simulink model "TosAeb"  (R2021a)
settings: Solver=FixedStepDiscrete  StartTime=0.0  StopTime=inf  FixedStep=0.01  RelTol=1e-3
undefined symbols (not defined in the file or in its folder): ACCModSts, AccSts1, AEB_ObjectMovingOrientation, AEB_ObjectMovingState, CN_BTCActive, CN_CurrentGearR, CN_CurrentGearR_DHT, CN_PTCActive, CN_VDCActive, CN_VSC_EspOff, CN_VSC_FCW_AEB_Error, CN_VSC_FCW_AEB_NoDispay, CN_VSC_FCW_AEB_PerfmDgdtn, CN_VSC_FCW_AEB_TempUnAvlbl, CN_VSC_VehStandstill, CNaccelAvoidMax, CNaccelAvoidMin, CNbrakePedalPress, CNcomfortStrLeftAccelMax, CNcomfortStrLeftAccelMin, CNcomfortStrRightAccelMax, CNcomfortStrRightAccelMin, CNDrvDoorOpen, CNDrvModeEco, CNDrvModeNorm, CNDrvModeOther, CNDrvModeSport, CNDrvSBRreminder, CNNull, CNobjInvalidID, CNPassDoorOpen, CNPassSBRreminder, CNPEBSAccelNegInf, CNPEBSAccelNull, CNPEBSTimeInf, CNPEBSTimeNull, CNSTOPMAX, CNSTOPNULL, CNsubjWidth, CNSwtOFF, CNSwtON, CNTTCMAX, CNTTCNULL, CNVehConfigAEBCycNone, CNVehConfigAEBNone, CNVehConfigAEBPedNone, CNVehConfigFCWNone, CNVehConfigJANone, CollisionSeverity, FALSE, FCWSnvty, JACycObjCalcInfoDefaultParams, JAPedObjCalcInfoDefaultParams, JAVehObjCalcInfoDefaultParams, latCycObjCalcInfoDefaultParams, latPedObjCalcInfoDefaultParams, longObjCalcInfoDefaultParams, ModSts, movingStatus, ObjectType ... (+1112)
libraries: simulink x3031, AEBSFunctionLib x50, Ta_Library x33, AEBSStateMachineLib x23, Mylibrary x12, simulink_extras x7, ObjSelect_lib x3, dspstat3 x2
(part 62 of 102)

== system TosAeb/FcwAebJa_Step_sys/ALG_AEBSystem/ALG_AEBController/AEB_LatObjInfoCalcApp/LatCycObjCalc/LatCycCollisonAssessInfoCalc/LatCycCollisonAssessInfoCalc/DistBrkCondCalc/CycDistBrakePartCalc/DistPhase3Calc ==
  egoStopFlg : Inport
  tJerkWork : Inport  Port=2  OutDataTypeStr=single
  tPhase2 : Inport  Port=3  OutDataTypeStr=single
  tPhase1 : Inport  Port=4  OutDataTypeStr=single
  velDelta : Inport  Port=5  OutDataTypeStr=single
  subjVel : Inport  Port=6
  subjLongAccelFilt : Inport  Port=7  OutDataTypeStr=single
  Kjerk : Inport  Port=8  OutDataTypeStr=single
  accelMax : Inport  Port=9  OutDataTypeStr=single
  Bus Creator : BusCreator  Inputs=8
  Bus Selector : BusSelector  OutputSignals=subjVel,subjLongAccelFilt,tJerkWork,Kjerk,accelMax,velDelta
  Bus Selector1 : BusSelector  OutputSignals=subjVel,subjLongAccelFilt,Kjerk,tPhase1,tPhase2,accelMax
  If : If  IfExpression=u1
  Merge : Merge
  distPhase3Calc : Subsystem
  tPhase3Calc : Subsystem  TreatAsAtomicUnit=on
  tPhase3Calc1 : Subsystem  TreatAsAtomicUnit=on
  distPhase3 : Outport
  wires:
  Bus Selector1/1 -> distPhase3Calc/2(subjVel)  "<subjVel>"
  Bus Selector1/2 -> distPhase3Calc/3(subjLongAccelFilt)  "<subjLongAccelFilt>"
  Bus Selector1/3 -> distPhase3Calc/4(Kjerk)  "<Kjerk>"
  Bus Selector1/4 -> distPhase3Calc/5(tPhase1)  "<tPhase1>"
  Bus Selector1/5 -> distPhase3Calc/6(tPhase2)  "<tPhase2>"
  Bus Selector1/6 -> distPhase3Calc/7(accelMax)  "<accelMax>"
  Bus Selector/6 -> tPhase3Calc/6(velDelta)  "<velDelta>"
  Bus Selector/1 -> tPhase3Calc/1(subjVel)  "<subjVel>"
  Bus Selector/5 -> tPhase3Calc/5(accelMax)  "<accelMax>"
  Bus Selector/2 -> tPhase3Calc/2(subjLongAccelFilt)  "<subjLongAccelFilt>"
  Bus Selector/4 -> tPhase3Calc/4(Kjerk)  "<Kjerk>"
  Bus Selector/3 -> tPhase3Calc/3(tJerkWork)  "<tJerkWork>"
  Bus Creator/1 -> Bus Selector/1, Bus Selector1/1
  velDelta/1 -> Bus Creator/4  "velDelta"
  tPhase2/1 -> Bus Creator/3  "tPhase2"
  tPhase1/1 -> Bus Creator/2  "tPhase1"
  tJerkWork/1 -> Bus Creator/1  "tJerkWork"
  subjVel/1 -> Bus Creator/5  "subjVel"
  subjLongAccelFilt/1 -> Bus Creator/6  "subjLongAccelFilt"
  Kjerk/1 -> Bus Creator/7  "Kjerk"
  accelMax/1 -> Bus Creator/8  "accelMax"
  distPhase3Calc/1(distPhase3) -> distPhase3/1
  Merge/1 -> distPhase3Calc/1(tPhase3)
  tPhase3Calc/1(tPhase3) -> Merge/2
  tPhase3Calc1/1(tPhase3) -> Merge/1
  If/2 -> tPhase3Calc/Action
  egoStopFlg/1 -> If/1
  If/1 -> tPhase3Calc1/Action

== system TosAeb/FcwAebJa_Step_sys/ALG_AEBSystem/ALG_AEBController/AEB_LatObjInfoCalcApp/LatCycObjCalc/LatCycCollisonAssessInfoCalc/LatCycCollisonAssessInfoCalc/DistBrkCondCalc/CycDistBrakePartCalc/distBrakeCalc ==
  distPhase1 : Inport  OutDataTypeStr=single
  distPhase2 : Inport  Port=2  OutDataTypeStr=single
  distPhase3 : Inport  Port=3  OutDataTypeStr=single
  Add : Sum  Inputs=3  OutDataTypeStr=single
  distBrake : Outport
  wires:
  Add/1 -> distBrake/1
  distPhase3/1 -> Add/3
  distPhase1/1 -> Add/1
  distPhase2/1 -> Add/2

== system TosAeb/FcwAebJa_Step_sys/ALG_AEBSystem/ALG_AEBController/AEB_LatObjInfoCalcApp/LatCycObjCalc/LatCycCollisonAssessInfoCalc/LatCycCollisonAssessInfoCalc/DistBrkCondCalc/distBraking4FullBrakeCalc1/DistPhase1Calc ==
  subjlongAccelCorr : Inport  OutDataTypeStr=single
  tdelay : Inport  Port=2  OutDataTypeStr=single
  subjVel : Inport  Port=3
  Add : Sum  OutDataTypeStr=single
  Gain : Gain  Gain=0.5  OutDataTypeStr=single
  Math Function : Math  Operator=square  SignedPower=on  OutDataTypeStr=single  SaturateOnIntegerOverflow=on
  Product : Product  OutDataTypeStr=single
  Product1 : Product  OutDataTypeStr=single
  distPhase1 : Outport
  timeWorkPhase1 : Outport  Port=2
  wires:
  Gain/1 -> Add/1
  Product/1 -> Add/2
  Add/1 -> distPhase1/1
  Product1/1 -> Gain/1
  subjlongAccelCorr/1 -> Product1/1
  Math Function/1 -> Product1/2
  subjVel/1 -> Product/2
  tdelay/1 -> Math Function/1, timeWorkPhase1/1, Product/1

== system TosAeb/FcwAebJa_Step_sys/ALG_AEBSystem/ALG_AEBController/AEB_LatObjInfoCalcApp/LatCycObjCalc/LatCycCollisonAssessInfoCalc/LatCycCollisonAssessInfoCalc/DistBrkCondCalc/distBraking4FullBrakeCalc1/DistPhase2Calc ==
  note: 增加输出接口4
  accelMax : Inport  OutDataTypeStr=single
  accelWorkPhase1 : Inport  Port=2  OutDataTypeStr=single
  Kjerk : Inport  Port=3  OutDataTypeStr=single
  subjVel : Inport  Port=4
  distPhase2Calc : Subsystem
  tWorkPhase2Calc : Subsystem
  distPhase2 : Outport
  Phase2EgoStopFlg : Outport  Port=2
  tWorkPhase2 : Outport  Port=3
  wires:
  [subjLongAccelCorr] -> distPhase2Calc/4(subjLongAccelCorr)
  [Kjerk] -> distPhase2Calc/3(Kjerk)
  accelWorkPhase1/1 -> [subjLongAccelCorr], tWorkPhase2Calc/2(accelWorkPhase1)
  accelMax/1 -> tWorkPhase2Calc/1(accelMax)
  Kjerk/1 -> [Kjerk], tWorkPhase2Calc/3(Kjerk)
  tWorkPhase2Calc/2(tWorkPhase2) -> tWorkPhase2/1, distPhase2Calc/1(tWorkPhase2)
  subjVel/1 -> tWorkPhase2Calc/4(subjVel), distPhase2Calc/2(subjVel)
  distPhase2Calc/1(distPhase2) -> distPhase2/1
  tWorkPhase2Calc/1(Phase2EgoStopFlg) -> Phase2EgoStopFlg/1

== system TosAeb/FcwAebJa_Step_sys/ALG_AEBSystem/ALG_AEBController/AEB_LatObjInfoCalcApp/LatCycObjCalc/LatCycCollisonAssessInfoCalc/LatCycCollisonAssessInfoCalc/DistBrkCondCalc/distBraking4FullBrakeCalc1/brkSysAtributeCalc ==
  subjVel : Inport
  subLongAccel : Inport  Port=2  OutDataTypeStr=single
  Bus Creator : BusCreator  Inputs=5
  Compare To Constant1 : simulink/Logic and Bit Operations/Compare To Constant  relop=>  const=11.94  OutDataTypeStr=boolean
  Constant : Constant  Value=0.5  OutDataTypeStr=single
  Constant2 : Constant  Value=-45  OutDataTypeStr=single
  Constant3 : Constant  Value=-10.5  OutDataTypeStr=single
  Constant4 : Constant  Value=-6.94  OutDataTypeStr=single
  Constant5 : Constant  Value=-11.11  OutDataTypeStr=single
  Min : MinMax  Function=max  Inputs=2  OutDataTypeStr=single
  Switch1 : Switch  Criteria=u2 ~= 0  OutDataTypeStr=single
  brkSysAtribute : Outport
  wires:
  Constant2/1 -> Bus Creator/2  "Kjerk"
  Bus Creator/1 -> brkSysAtribute/1
  Compare To Constant1/1 -> Switch1/2
  subjVel/1 -> Compare To Constant1/1
  Constant4/1 -> Switch1/1
  Constant5/1 -> Switch1/3
  Constant/1 -> Bus Creator/1  "tdelay"
  Constant3/1 -> Min/2, Bus Creator/4  "accelMax"
  Switch1/1 -> Bus Creator/5  "velReduceMax"
  subLongAccel/1 -> Min/1
  Min/1 -> Bus Creator/3  "subjLongAccelCorr"

== system TosAeb/FcwAebJa_Step_sys/ALG_AEBSystem/ALG_AEBController/AEB_LatObjInfoCalcApp/LatCycObjCalc/LatCycCollisonAssessInfoCalc/LatCycCollisonAssessInfoCalc/DistBrkCondCalc/distBraking4FullBrakeCalc1/distPhase3Calc ==
  Phase2EgoStopFlg : Inport
  tWorkPhase2 : Inport  Port=2  OutDataTypeStr=single
  subjLongAccelCorr : Inport  Port=3  OutDataTypeStr=single
  Kjerk : Inport  Port=4  OutDataTypeStr=single
  accelWorkPhase1 : Inport  Port=5  OutDataTypeStr=single
  velMaxReduce : Inport  Port=6  OutDataTypeStr=single
  accelMax : Inport  Port=7  OutDataTypeStr=single
  tWorkPhase1 : Inport  Port=8  OutDataTypeStr=single
  EgoStopped : Subsystem  TreatAsAtomicUnit=on
  If : If  IfExpression=u1
  Merge : Merge
  distPhase3Calc : Subsystem  TreatAsAtomicUnit=on
  distPhase3 : Outport
  wires:
  tWorkPhase1/1 -> distPhase3Calc/7(tWorkPhase1)
  accelMax/1 -> distPhase3Calc/6(accelMax)
  velMaxReduce/1 -> distPhase3Calc/5(velMaxReduce)
  accelWorkPhase1/1 -> distPhase3Calc/3(accelWorkPhase1)
  subjLongAccelCorr/1 -> distPhase3Calc/4(subjLongAccelCorr)
  tWorkPhase2/1 -> distPhase3Calc/2(tWorkPhase2)
  Kjerk/1 -> distPhase3Calc/1(Kjerk)
  Phase2EgoStopFlg/1 -> If/1
  If/2 -> distPhase3Calc/Action
  EgoStopped/1(distPhase3) -> Merge/1
  If/1 -> EgoStopped/Action
  Merge/1 -> distPhase3/1
  distPhase3Calc/1(distPhase3) -> Merge/2

== system TosAeb/FcwAebJa_Step_sys/ALG_AEBSystem/ALG_AEBController/AEB_LatObjInfoCalcApp/LatCycObjCalc/LatCycCollisonAssessInfoCalc/LatCycCollisonAssessInfoCalc/DistBrkCondCalc/distBraking4FullBrakeCalc1/distanceTriggerBrakingCalc ==
  distPhase1 : Inport  OutDataTypeStr=single
  distPhase2 : Inport  Port=2  OutDataTypeStr=single
  distPhase3 : Inport  Port=3  OutDataTypeStr=single
  Add : Sum  Inputs=+++  OutDataTypeStr=single
  DistBraking4FullBrake : Outport
  wires:
  Add/1 -> DistBraking4FullBrake/1
  distPhase3/1 -> Add/3
  distPhase2/1 -> Add/2
  distPhase1/1 -> Add/1

== system TosAeb/FcwAebJa_Step_sys/ALG_AEBSystem/ALG_AEBController/AEB_LatObjInfoCalcApp/LatCycObjCalc/LatCycCollisonAssessInfoCalc/LatCycCollisonAssessInfoCalc/DistBrkCondCalc/distBraking4PartBrakeCalc1/DistPhase1Calc ==
  subjlongAccelCorr : Inport  OutDataTypeStr=single
  tdelay : Inport  Port=2  OutDataTypeStr=single
  subjVel : Inport  Port=3
  Add : Sum  OutDataTypeStr=single
  Gain : Gain  Gain=0.5  OutDataTypeStr=single
  Math Function : Math  Operator=square  SignedPower=on  OutDataTypeStr=single  SaturateOnIntegerOverflow=on
  Product : Product  OutDataTypeStr=single
  Product1 : Product  OutDataTypeStr=single
  distPhase1 : Outport
  timeWorkPhase1 : Outport  Port=2
  wires:
  tdelay/1 -> Product/1, timeWorkPhase1/1, Math Function/1
  subjVel/1 -> Product/2
  Math Function/1 -> Product1/2
  subjlongAccelCorr/1 -> Product1/1
  Product1/1 -> Gain/1
  Add/1 -> distPhase1/1
  Product/1 -> Add/2
  Gain/1 -> Add/1

== system TosAeb/FcwAebJa_Step_sys/ALG_AEBSystem/ALG_AEBController/AEB_LatObjInfoCalcApp/LatCycObjCalc/LatCycCollisonAssessInfoCalc/LatCycCollisonAssessInfoCalc/DistBrkCondCalc/distBraking4PartBrakeCalc1/DistPhase2Calc ==
  note: 增加输出接口4
  accelMax : Inport  OutDataTypeStr=single
  accelWorkPhase1 : Inport  Port=2  OutDataTypeStr=single
  Kjerk : Inport  Port=3  OutDataTypeStr=single
  subjVel : Inport  Port=4
  distPhase2Calc : Subsystem
  tWorkPhase2Calc : Subsystem
  distPhase2 : Outport
  Phase2EgoStopFlg : Outport  Port=2
  tWorkPhase2 : Outport  Port=3
  wires:
  tWorkPhase2Calc/1(Phase2EgoStopFlg) -> Phase2EgoStopFlg/1
  distPhase2Calc/1(distPhase2) -> distPhase2/1
  subjVel/1 -> distPhase2Calc/2(subjVel), tWorkPhase2Calc/4(subjVel)
  tWorkPhase2Calc/2(tWorkPhase2) -> distPhase2Calc/1(tWorkPhase2), tWorkPhase2/1
  Kjerk/1 -> tWorkPhase2Calc/3(Kjerk), [Kjerk]
  accelMax/1 -> tWorkPhase2Calc/1(accelMax)
  accelWorkPhase1/1 -> tWorkPhase2Calc/2(accelWorkPhase1), [subjLongAccelCorr]
  [Kjerk] -> distPhase2Calc/3(Kjerk)
  [subjLongAccelCorr] -> distPhase2Calc/4(subjLongAccelCorr)

== system TosAeb/FcwAebJa_Step_sys/ALG_AEBSystem/ALG_AEBController/AEB_LatObjInfoCalcApp/LatCycObjCalc/LatCycCollisonAssessInfoCalc/LatCycCollisonAssessInfoCalc/DistBrkCondCalc/distBraking4PartBrakeCalc1/brkSysAtributeCalc ==
  subjVel : Inport
  subLongAccel : Inport  Port=2  OutDataTypeStr=single
  Bus Creator : BusCreator  Inputs=5
  Compare To Constant1 : simulink/Logic and Bit Operations/Compare To Constant  relop=>  const=11.94  OutDataTypeStr=boolean
  Constant : Constant  Value=0.5  OutDataTypeStr=single
  Constant2 : Constant  Value=-45  OutDataTypeStr=single
  Constant3 : Constant  Value=-5.75  OutDataTypeStr=single
  Constant4 : Constant  Value=-6.94  OutDataTypeStr=single
  Constant5 : Constant  Value=-8.3  OutDataTypeStr=single
  Min : MinMax  Function=max  Inputs=2  OutDataTypeStr=single
  Switch1 : Switch  Criteria=u2 ~= 0  OutDataTypeStr=single
  brkSysAtribute : Outport
  wires:
  Min/1 -> Bus Creator/3  "subjLongAccelCorr"
  subLongAccel/1 -> Min/1
  Switch1/1 -> Bus Creator/5  "velReduceMax"
  Constant3/1 -> Bus Creator/4, Min/2  "accelMax"
  Constant/1 -> Bus Creator/1  "tdelay"
  Constant5/1 -> Switch1/3
  Constant4/1 -> Switch1/1
  subjVel/1 -> Compare To Constant1/1
  Compare To Constant1/1 -> Switch1/2
  Bus Creator/1 -> brkSysAtribute/1
  Constant2/1 -> Bus Creator/2  "Kjerk"

== system TosAeb/FcwAebJa_Step_sys/ALG_AEBSystem/ALG_AEBController/AEB_LatObjInfoCalcApp/LatCycObjCalc/LatCycCollisonAssessInfoCalc/LatCycCollisonAssessInfoCalc/DistBrkCondCalc/distBraking4PartBrakeCalc1/distPhase3Calc ==
  Phase2EgoStopFlg : Inport
  tWorkPhase2 : Inport  Port=2  OutDataTypeStr=single
  subjLongAccelCorr : Inport  Port=3  OutDataTypeStr=single
  Kjerk : Inport  Port=4  OutDataTypeStr=single
  accelWorkPhase1 : Inport  Port=5  OutDataTypeStr=single
  velMaxReduce : Inport  Port=6  OutDataTypeStr=single
  accelMax : Inport  Port=7  OutDataTypeStr=single
  tWorkPhase1 : Inport  Port=8  OutDataTypeStr=single
  EgoStopped : Subsystem  TreatAsAtomicUnit=on
  If : If  IfExpression=u1
  Merge : Merge
  distPhase3Calc : Subsystem  TreatAsAtomicUnit=on
  distPhase3 : Outport
  wires:
  distPhase3Calc/1(distPhase3) -> Merge/2
  Merge/1 -> distPhase3/1
  If/1 -> EgoStopped/Action
  EgoStopped/1(distPhase3) -> Merge/1
  If/2 -> distPhase3Calc/Action
  Phase2EgoStopFlg/1 -> If/1
  Kjerk/1 -> distPhase3Calc/1(Kjerk)
  tWorkPhase2/1 -> distPhase3Calc/2(tWorkPhase2)
  subjLongAccelCorr/1 -> distPhase3Calc/4(subjLongAccelCorr)
  accelWorkPhase1/1 -> distPhase3Calc/3(accelWorkPhase1)
  velMaxReduce/1 -> distPhase3Calc/5(velMaxReduce)
  accelMax/1 -> distPhase3Calc/6(accelMax)
  tWorkPhase1/1 -> distPhase3Calc/7(tWorkPhase1)

== system TosAeb/FcwAebJa_Step_sys/ALG_AEBSystem/ALG_AEBController/AEB_LatObjInfoCalcApp/LatCycObjCalc/LatCycCollisonAssessInfoCalc/LatCycCollisonAssessInfoCalc/DistBrkCondCalc/distBraking4PartBrakeCalc1/distanceTriggerBrakingCalc ==
  distPhase1 : Inport  OutDataTypeStr=single
  distPhase2 : Inport  Port=2  OutDataTypeStr=single
  distPhase3 : Inport  Port=3  OutDataTypeStr=single
  Add : Sum  Inputs=+++  OutDataTypeStr=single
  distBraking4PartBrake : Outport
  wires:
  distPhase1/1 -> Add/1
  distPhase2/1 -> Add/2
  distPhase3/1 -> Add/3
  Add/1 -> distBraking4PartBrake/1

== system TosAeb/FcwAebJa_Step_sys/ALG_AEBSystem/ALG_AEBController/AEB_LatObjInfoCalcApp/LatCycObjCalc/LatCycCollisonAssessInfoCalc/LatCycCollisonAssessInfoCalc/DistBrkCondCalc/distSecurCalc/If Action Subsystem ==
  subjVel : Inport
  Action Port : ActionPort  ActionPortLabel=else
  1-D Lookup Table : Lookup_n-D  NumberOfTableDimensions=1  BreakpointsForDimension1=P_AEB_LatCycCA_SubjVelDxSecureUnder40KphX  BeginIndexSearchUsingPreviousIndexResult=on  Table=P_AEB_LatCycCA_DxSecureY  ExtrapMethod=Clip  InternalRulePriority=Speed  RndMeth=Simplest  InputSameDT=on
  distSecure : Outport
  wires:
  1-D Lookup Table/1 -> distSecure/1
  subjVel/1 -> 1-D Lookup Table/1

== system TosAeb/FcwAebJa_Step_sys/ALG_AEBSystem/ALG_AEBController/AEB_LatObjInfoCalcApp/LatCycObjCalc/LatCycCollisonAssessInfoCalc/LatCycCollisonAssessInfoCalc/DistBrkCondCalc/distSecurCalc/If Action Subsystem1 ==
  Action Port : ActionPort  ActionPortLabel=if(u1)
  Constant : Constant  Value=CNNull
  distSecure : Outport
  wires:
  Constant/1 -> distSecure/1

== system TosAeb/FcwAebJa_Step_sys/ALG_AEBSystem/ALG_AEBController/AEB_LatObjInfoCalcApp/LatCycObjCalc/LatCycObjCalc/latCycObjInfoCalc/ObjColiisionRiskProbCalc/CollisionRiskProbCalc/CollisionRiskProbCalc ==
  dxReginEgoRUCorr : Inport
  dyReginEgoRUCorr : Inport  Port=2
  dyReginEgoLLCorr : Inport  Port=3
  dxReginEgoLLCorr : Inport  Port=4
  dxReginObjRUCorr : Inport  Port=5
  dyReginObjRUCorr : Inport  Port=6
  dxReginObjLLCorr : Inport  Port=7
  dyReginObjLLCorr : Inport  Port=8
  Bus Creator : BusCreator  Inputs=8
  Bus Selector : BusSelector  OutputSignals=dyReginEgoRUCorr,dyReginEgoLLCorr,dyReginObjRUCorr,dyReginObjLLCorr
  Bus Selector1 : BusSelector  OutputSignals=dxReginEgoRUCorr,dxReginEgoLLCorr,dxReginObjRUCorr,dxReginObjLLCorr
  Bus Selector2 : BusSelector  OutputSignals=dxReginObjLLCorr,dyReginObjRUCorr,dyReginObjLLCorr
  CriticalityPointCalc : Subsystem
  dxOverlapFlow : Stateflow chart  TreatAsAtomicUnit=on
  dyOverlapFlow : Stateflow chart  TreatAsAtomicUnit=on
  pedCollisionRiskProbCalc : Subsystem
  dxPreCollison : Outport
  dyPreCollison : Outport  Port=2
  collisionRiskProb : Outport  Port=3
  dyCollisionRiskProb : Outport  Port=4
  dxCollisionRiskProb : Outport  Port=5
  wires:
  pedCollisionRiskProbCalc/3(dxCollisionRiskProb) -> dxCollisionRiskProb/1
  pedCollisionRiskProbCalc/2(dyCollisionRiskProb) -> dyCollisionRiskProb/1
  Bus Selector1/4 -> dxOverlapFlow/4(dxReginObjLLCorr)  "<dxReginObjLLCorr>"
  Bus Selector1/3 -> dxOverlapFlow/3(dxReginObjRUCorr)  "<dxReginObjRUCorr>"
  Bus Selector/4 -> dyOverlapFlow/4(dyReginObjLLCorr)  "<dyReginObjLLCorr>"
  Bus Selector/3 -> dyOverlapFlow/3(dyReginObjRUCorr)  "<dyReginObjRUCorr>"
  CriticalityPointCalc/2(dyPreCollison) -> dyPreCollison/1
  CriticalityPointCalc/1(dxPreCollison) -> dxPreCollison/1
  Bus Selector2/3 -> CriticalityPointCalc/3(dyReginObjLLCorr)  "<dyReginObjLLCorr>"
  Bus Selector2/2 -> CriticalityPointCalc/2(dyReginObjRUCorr)  "<dyReginObjRUCorr>"
  Bus Selector2/1 -> CriticalityPointCalc/1(dxReginObjLLCorr)  "<dxReginObjLLCorr>"
  Bus Selector1/2 -> dxOverlapFlow/2(dxReginEgoLLCorr)  "<dxReginEgoLLCorr>"
  Bus Selector1/1 -> dxOverlapFlow/1(dxReginEgoRUCorr)  "<dxReginEgoRUCorr>"
  Bus Selector/2 -> dyOverlapFlow/2(dyReginEgoLLCorr)  "<dyReginEgoLLCorr>"
  Bus Selector/1 -> dyOverlapFlow/1(dyReginEgoRUCorr)  "<dyReginEgoRUCorr>"
  Bus Creator/1 -> Bus Selector2/1, Bus Selector/1, Bus Selector1/1
  dyReginObjLLCorr/1 -> Bus Creator/8  "dyReginObjLLCorr"
  dxReginObjLLCorr/1 -> Bus Creator/7  "dxReginObjLLCorr"
  dyReginObjRUCorr/1 -> Bus Creator/6  "dyReginObjRUCorr"
  dxReginEgoRUCorr/1 -> Bus Creator/1  "dxReginEgoRUCorr"
  dxReginObjRUCorr/1 -> Bus Creator/5  "dxReginObjRUCorr"
  dxReginEgoLLCorr/1 -> Bus Creator/4  "dxReginEgoLLCorr"
  dyReginEgoRUCorr/1 -> Bus Creator/2  "dyReginEgoRUCorr"
  dyReginEgoLLCorr/1 -> Bus Creator/3  "dyReginEgoLLCorr"
  pedCollisionRiskProbCalc/1(collisionRiskProb) -> collisionRiskProb/1
  dxOverlapFlow/2(dxOverlapLimit) -> pedCollisionRiskProbCalc/4(dxOverlapLimit)
  dxOverlapFlow/1(dxOverlap) -> pedCollisionRiskProbCalc/3(dxOverlap)
  dyOverlapFlow/2(dyOverlapLimit) -> pedCollisionRiskProbCalc/2(dyOverlapLimit)
  dyOverlapFlow/1(dyOverlap) -> pedCollisionRiskProbCalc/1(dyOverlap)

== system TosAeb/FcwAebJa_Step_sys/ALG_AEBSystem/ALG_AEBController/AEB_LatObjInfoCalcApp/LatCycObjCalc/LatCycObjCalc/latCycObjInfoCalc/ObjColiisionRiskProbCalc/CollisionRiskProbCalc/EgoReginCalc ==
  tPredTime : Inport
  subjVel : Inport  Port=2
  Abs : Abs
  Add : Sum
  Add1 : Sum  Inputs=+-
  Constant : Constant  Value=P_Global_DxvRearBumper_m
  Constant1 : Constant  Value=P_Global_VehicleWidthMin_m
  Constant2 : Constant  Value=P_Global_DxvFrontBumper_m
  Gain : Gain  Gain=-0.5
  Gain1 : Gain  Gain=0.5
  Product : Product
  dxReginEgoRU : Outport
  dyReginEgoRU : Outport  Port=2
  dxReginEgoLL : Outport  Port=3
  dyReginEgoLL : Outport  Port=4
  wires:
  subjVel/1 -> Product/2
  tPredTime/1 -> Product/1
  Add1/1 -> dxReginEgoLL/1
  Constant/1 -> Abs/1
  Gain1/1 -> dyReginEgoLL/1
  Gain/1 -> dyReginEgoRU/1
  Constant1/1 -> Gain/1, Gain1/1
  Add/1 -> dxReginEgoRU/1
  Constant2/1 -> Add/2
  Product/1 -> Add1/1, Add/1
  Abs/1 -> Add1/2

== system TosAeb/FcwAebJa_Step_sys/ALG_AEBSystem/ALG_AEBController/AEB_LatObjInfoCalcApp/LatCycObjCalc/LatCycObjCalc/latCycObjInfoCalc/ObjColiisionRiskProbCalc/CollisionRiskProbCalc/EgoReginCorrCalc ==
  dxReginEgoRU : Inport
  dyReginEgoRU : Inport  Port=2
  dxReginEgoLL : Inport  Port=3
  dyReginEgoLL : Inport  Port=4
  Add : Sum  OutDataTypeStr=single
  Add1 : Sum  Inputs=+-  OutDataTypeStr=single
  Add2 : Sum  OutDataTypeStr=single
  ObjectLength : Constant  Value=0.5  OutDataTypeStr=single
  ObjectWidth : Constant  Value=1.8  OutDataTypeStr=single
  dxReginEgoRUCorr : Outport
  dyReginEgoRUCorr : Outport  Port=2
  dyReginEgoLLCorr : Outport  Port=3
  dxReginEgoLLCorr : Outport  Port=4
  wires:
  ObjectLength/1 -> Add/1
  dxReginEgoRU/1 -> Add/2
  Add2/1 -> dyReginEgoLLCorr/1
  dyReginEgoLL/1 -> Add2/2
  dxReginEgoLL/1 -> dxReginEgoLLCorr/1
  Add1/1 -> dyReginEgoRUCorr/1
  ObjectWidth/1 -> Add1/2, Add2/1
  dyReginEgoRU/1 -> Add1/1
  Add/1 -> dxReginEgoRUCorr/1

== system TosAeb/FcwAebJa_Step_sys/ALG_AEBSystem/ALG_AEBController/AEB_LatObjInfoCalcApp/LatCycObjCalc/LatCycObjCalc/latCycObjInfoCalc/ObjColiisionRiskProbCalc/CollisionRiskProbCalc/ObjAbsInfoCalc ==
  vx : Inport
  vy : Inport  Port=2
  dy : Inport  Port=3
  dxFrontBumper : Inport  Port=4
  subjYawRate : Inport  Port=5
  subjVel : Inport  Port=6
  Bus Creator1 : BusCreator  Inputs=2
  RadicalRelevantCalc : Subsystem
  RadicalToXYTrans : Subsystem
  XYToRadicalTrans : Subsystem
  sincosCoefCalc : Subsystem
  velAbsObjCorr : Outport
  wires:
  RadicalToXYTrans/1(velAbsObjCorr) -> velAbsObjCorr/1
  RadicalRelevantCalc/2(objAngleToRear) -> sincosCoefCalc/1(angle)  "objAngleToRear"
  XYToRadicalTrans/1(velRadicalXY) -> RadicalToXYTrans/2(velRadicalXY)
  vy/1 -> Bus Creator1/2  "vyRelTargetObjCalc"
  Bus Creator1/1 -> XYToRadicalTrans/1(LongLatVelRel)  "LongLatVelRel"
  dxFrontBumper/1 -> RadicalRelevantCalc/2(dxTargetObjCalc)
  subjVel/1 -> RadicalToXYTrans/1(subjVel)
  vx/1 -> Bus Creator1/1  "vxRelTargetObjCalc"
  sincosCoefCalc/1(sincosCoef) -> RadicalToXYTrans/3(sincosCoef), XYToRadicalTrans/3(sincosCoef)
  dy/1 -> RadicalRelevantCalc/1(dyTargetObjCalc)
  subjYawRate/1 -> RadicalRelevantCalc/3(subjYawRate)
  RadicalRelevantCalc/1(radialVelRel) -> XYToRadicalTrans/2(radialVelRel)  "radialVelRel"

== system TosAeb/FcwAebJa_Step_sys/ALG_AEBSystem/ALG_AEBController/AEB_LatObjInfoCalcApp/LatCycObjCalc/LatCycObjCalc/latCycObjInfoCalc/ObjColiisionRiskProbCalc/CollisionRiskProbCalc/ObjReginCorrCalc ==
  velAbsObjCorr : Inport
  tPredTime : Inport  Port=2
  ObjectTypeCameraConfirmed : Inport  Port=3
  ObjectWidth : Inport  Port=4
  ObjectYawAngle : Inport  Port=5
  ObjectMovingOrientation : Inport  Port=6
  ObjectLength : Inport  Port=7
  dx : Inport  Port=8
  dy : Inport  Port=9
  vxAbsObj : Inport  Port=10
  vy : Inport  Port=11
  AccelXYMaxMinCorrCalc : Subsystem
  CycMaxAccelDecelCalc : Subsystem
  ObjFrameCalc : Subsystem
  ObjLengthWidthCalc : Subsystem
  ObjLengthWidthCalc1 : Subsystem  Commented=on
  ObjReginCorrCalc : Subsystem
  dxReginObjRUCorr : Outport
  dyReginObjRUCorr : Outport  Port=2
  dxReginObjLLCorr : Outport  Port=3
  dyReginObjLLCorr : Outport  Port=4
  wires:
  ObjReginCorrCalc/4(dyReginObjLLCorr) -> dyReginObjLLCorr/1
  ObjReginCorrCalc/3(dxReginObjLLCorr) -> dxReginObjLLCorr/1
  ObjReginCorrCalc/2(dyReginObjRUCorr) -> dyReginObjRUCorr/1
  ObjReginCorrCalc/1(dxReginObjRUCorr) -> dxReginObjRUCorr/1
  ObjLengthWidthCalc/2(ObjWidthCorr) -> ObjFrameCalc/4(ObjWidthCorr)
  ObjLengthWidthCalc/1(ObjLengthCorr) -> ObjFrameCalc/3(ObjLengthCorr)
  ObjectLength/1 -> ObjLengthWidthCalc/2(ObjectLength)
  ObjectYawAngle/1 -> ObjLengthWidthCalc/4(ObjectYawAngle)
  ObjectWidth/1 -> ObjLengthWidthCalc/3(ObjectWidth)
  dy/1 -> ObjFrameCalc/2(dy)
  dx/1 -> ObjFrameCalc/1(dx)
  ObjFrameCalc/4(dyMin) -> ObjReginCorrCalc/7(dyMin)
  ObjFrameCalc/2(dxMin) -> ObjReginCorrCalc/5(dxMin)
  AccelXYMaxMinCorrCalc/2(AccelYMaxMinCorr) -> ObjReginCorrCalc/2(AccelYMaxMinCorr)
  ObjFrameCalc/3(dyMax) -> ObjReginCorrCalc/6(dyMax)
  ObjFrameCalc/1(dxMax) -> ObjReginCorrCalc/4(dxMax)
  CycMaxAccelDecelCalc/1(maxAccelDecel) -> AccelXYMaxMinCorrCalc/1(maxAccelDecel)
  velAbsObjCorr/1 -> ObjReginCorrCalc/3(velAbsTargetCorr), AccelXYMaxMinCorrCalc/2(velAbsTargetCorr)
  AccelXYMaxMinCorrCalc/1(AccelXMaxMinCorr) -> ObjReginCorrCalc/1(AccelXMaxMinCorr)
  tPredTime/1 -> ObjReginCorrCalc/8(tPredTime)
  vxAbsObj/1 -> ObjReginCorrCalc/9(vxAbsObj)
  vy/1 -> ObjReginCorrCalc/10(vy)
  ObjectMovingOrientation/1 -> ObjLengthWidthCalc/5(ObjectMovingOrientation)
  ObjectTypeCameraConfirmed/1 -> ObjLengthWidthCalc/1(ObjectTypeCameraConfirmed)

== system TosAeb/FcwAebJa_Step_sys/ALG_AEBSystem/ALG_AEBController/AEB_LatObjInfoCalcApp/LatCycObjCalc/LatCycObjCalc/latCycObjInfoCalc/ObjCrossFlgCal/CycPreCollisionCalcFunc/ObjBoundingBox ==
  ObjLengthCorr : Inport  OutDataTypeStr=single
  ObjWidthCorr : Inport  Port=2  OutDataTypeStr=single
  dxPreRefPointObj : Inport  Port=3  OutDataTypeStr=single
  dyPreRefPointObj : Inport  Port=4  OutDataTypeStr=single
  sincosCoef : Inport  Port=5
  Bus Creator : BusCreator  Inputs=5
  Mux : Mux
  Mux1 : Mux
  ObjLLCoordinate : Subsystem
  ObjLUCoordinate : Subsystem
  ObjRLCoordinate : Subsystem
  ObjRUCoordinate : Subsystem
  objDxPre : Outport
  objDyPre : Outport  Port=2
  wires:
  [dyPreRLObj] -> Mux1/4  "dyPreRLObj"
  [dyPreLLObj] -> Mux1/3  "dyPreLLObj"
  [dyPreLUObj] -> Mux1/2  "dyPreLUObj"
  [dyPreRUObj] -> Mux1/1  "dyPreRUObj"
  [dxPreRLObj] -> Mux/4  "dxPreRLObj"
  [dxPreLLObj] -> Mux/3  "dxPreLLObj"
  [dxPreLUObj] -> Mux/2  "dxPreLUObj"
  [dxPreRUObj] -> Mux/1  "dxPreRUObj"
  Mux1/1 -> objDyPre/1
  Mux/1 -> objDxPre/1
  ObjRLCoordinate/2(dyPreRLObj) -> [dyPreRLObj]  "dyPreRLObj"
  ObjRLCoordinate/1(dxPreRLObj) -> [dxPreRLObj]  "dxPreRLObj"
  ObjRUCoordinate/2(dyPreRUObj) -> [dyPreRUObj]  "dyPreRUObj"
  ObjRUCoordinate/1(dxPreRUObj) -> [dxPreRUObj]  "dxPreRUObj"
  ObjLLCoordinate/2(dyPreLLObj) -> [dyPreLLObj]  "dyPreLLObj"
  ObjLLCoordinate/1(dxPre_LLObj) -> [dxPreLLObj]  "dxPreLLObj"
  ObjLUCoordinate/2(dyPreLUObj) -> [dyPreLUObj]  "dyPreLUObj"
  ObjLUCoordinate/1(dxPreLUObj) -> [dxPreLUObj]  "dxPreLUObj"
  Bus Creator/1 -> ObjLUCoordinate/1(inputSignals), ObjLLCoordinate/1(inputSignals), ObjRUCoordinate/1(inputSignals), ObjRLCoordinate/1(inputSignals)
  sincosCoef/1 -> Bus Creator/5  "sincosCoef"
  dyPreRefPointObj/1 -> Bus Creator/4  "dyPreRefPointObj"
  dxPreRefPointObj/1 -> Bus Creator/3  "dxPreRefPointObj"
  ObjWidthCorr/1 -> Bus Creator/2  "ObjWidthCorr"
  ObjLengthCorr/1 -> Bus Creator/1  "ObjLengthCorr"

== system TosAeb/FcwAebJa_Step_sys/ALG_AEBSystem/ALG_AEBController/AEB_LatObjInfoCalcApp/LatCycObjCalc/LatCycObjCalc/latCycObjInfoCalc/ObjCrossFlgCal/CycPreCollisionCalcFunc/ObjLenthWidthCalc ==
  ObjectTypeCameraConfirmed : Inport
  ObjectLength : Inport  Port=2
  ObjectWidth : Inport  Port=3
  ObjectYawAngle : Inport  Port=4
  ObjectMovingOrientation : Inport  Port=5
  ObjLengthWidthCalc : Stateflow chart  Commented=on  TreatAsAtomicUnit=on
  ObjLengthWidthCalc1 : Stateflow chart  TreatAsAtomicUnit=on
  P_LatCycCA_PreCollisionCycLengthThres : Constant  Value=P_AEB_LatCycCA_PreCollisionCycLengthThres
  P_LatCycCA_PreCollisionCycWidthThres : Constant  Value=P_AEB_LatCycCA_PreCollisionCycWidthThres
  ObjLengthCorr : Outport
  ObjWidthCorr : Outport  Port=2
  wires:
  ObjLengthWidthCalc1/2(ObjectWidth) -> ObjWidthCorr/1
  ObjLengthWidthCalc1/1(ObjectLength) -> ObjLengthCorr/1
  P_LatCycCA_PreCollisionCycLengthThres/1 -> ObjLengthWidthCalc1/6(P_LatCycCA_PreCollisionCycLengthThres)
  P_LatCycCA_PreCollisionCycWidthThres/1 -> ObjLengthWidthCalc1/7(P_LatCycCA_PreCollisionCycWidthThres)
  ObjectMovingOrientation/1 -> ObjLengthWidthCalc1/5(ObjectMovingOrientation)
  ObjectYawAngle/1 -> ObjLengthWidthCalc1/4(ObjectYawAngle)
  ObjectWidth/1 -> ObjLengthWidthCalc1/3(ObjectWidthIn)
  ObjectLength/1 -> ObjLengthWidthCalc1/2(ObjectLengthIn)
  ObjectTypeCameraConfirmed/1 -> ObjLengthWidthCalc1/1(ObjectTypeCameraConfirmed)

== system TosAeb/FcwAebJa_Step_sys/ALG_AEBSystem/ALG_AEBController/AEB_LatObjInfoCalcApp/LatCycObjCalc/LatCycObjCalc/latCycObjInfoCalc/ObjCrossFlgCal/CycPreCollisionCalcFunc/ObjPreReferPointCalc ==
  dx : Inport
  vxAbsObj : Inport  Port=2
  tPredTime : Inport  Port=3  OutDataTypeStr=single
  axAbsObj : Inport  Port=4
  vy : Inport  Port=5
  subjLatAccel : Inport  Port=6
  ay : Inport  Port=7
  dy : Inport  Port=8
  ObjectYawAngle : Inport  Port=9
  Add : Sum  Inputs=+++  OutDataTypeStr=single
  Add1 : Sum  Inputs=+++  OutDataTypeStr=single
  Add2 : Sum
  Degrees to Radians : simulink_extras/Transformations/Degrees to Radians
  Gain : Gain  Gain=0.5  OutDataTypeStr=single
  Gain1 : Gain  Gain=0.5  OutDataTypeStr=single
  Math Function : Math  Operator=square  SignedPower=on  OutDataTypeStr=single  SaturateOnIntegerOverflow=on
  Math Function1 : Math  Operator=square  SignedPower=on  OutDataTypeStr=single  SaturateOnIntegerOverflow=on
  Product{,1..3} (4 alike) : Product  OutDataTypeStr=single
  dxPreRefPointObj : Outport
  dyPreRefPointObj : Outport  Port=2
  YawAnglePreObj : Outport  Port=3
  wires:
  Add2/1 -> Product3/2
  ay/1 -> Add2/2
  subjLatAccel/1 -> Add2/1
  ObjectYawAngle/1 -> Degrees to Radians/1
  Add1/1 -> dyPreRefPointObj/1
  dy/1 -> Add1/3
  Gain1/1 -> Add1/2
  Product2/1 -> Add1/1
  Product3/1 -> Gain1/1
  Math Function1/1 -> Product3/1
  vy/1 -> Product2/1
  dx/1 -> Add/1
  Add/1 -> dxPreRefPointObj/1
  Gain/1 -> Add/3
  Product1/1 -> Gain/1
  axAbsObj/1 -> Product1/2
  Math Function/1 -> Product1/1
  Product/1 -> Add/2
  tPredTime/1 -> Product2/2, Math Function1/1, Math Function/1, Product/2
  vxAbsObj/1 -> Product/1
  Degrees to Radians/1 -> YawAnglePreObj/1

== system TosAeb/FcwAebJa_Step_sys/ALG_AEBSystem/ALG_AEBController/AEB_LatObjInfoCalcApp/LatCycObjCalc/LatCycObjCalc/latCycObjInfoCalc/ObjCrossFlgCal/CycPreCollisionCalcFunc/PreCrossFlgCalcFlow/VectorCrossAlgorithm ==
  x1 : Inport  OutDataTypeStr=single
  y1 : Inport  Port=2  OutDataTypeStr=single
  x2 : Inport  Port=3  OutDataTypeStr=single
  y2 : Inport  Port=4  OutDataTypeStr=single
  m1 : Inport  Port=5  OutDataTypeStr=single
  n1 : Inport  Port=6  OutDataTypeStr=single
  m2 : Inport  Port=7  OutDataTypeStr=single
  n2 : Inport  Port=8  OutDataTypeStr=single
  f : TriggerPort  TriggerType=function-call  VariantControl=Variant
      settings: CopyFcn=Stateflow.SLINSF.SimulinkMan.removeBlockPreDeleteFcn  PreDeleteFcn=Stateflow.SLINSF.SimulinkMan.unsafePredeleteFcn
  Abs : Abs
  Bus Creator : BusCreator  Inputs=8
  Bus Selector : BusSelector  OutputSignals=x2,x1,n2,n1,y2,y1,m2,m1
  Compare To Constant : simulink/Logic and Bit Operations/Compare To Constant  relop=<  const=1e-6  OutDataTypeStr=boolean
  If : If  IfExpression=u1
  If Action Subsystem : Subsystem  TreatAsAtomicUnit=on
  If Action Subsystem1 : Subsystem  TreatAsAtomicUnit=on
  Merge : Merge
  VectorMulit : Subsystem
  pedPreCollisionFlg : Outport
  wires:
  Bus Selector/8 -> VectorMulit/8(m1)  "<m1>"
  Bus Selector/7 -> VectorMulit/7(m2)  "<m2>"
  Bus Selector/6 -> VectorMulit/6(y1)  "<y1>"
  Bus Selector/5 -> VectorMulit/5(y2)  "<y2>"
  Bus Selector/4 -> VectorMulit/4(n1)  "<n1>"
  Bus Selector/3 -> VectorMulit/3(n2)  "<n2>"
  Bus Selector/1 -> VectorMulit/1(x2)  "<x2>"
  Bus Selector/2 -> VectorMulit/2(x1)  "<x1>"
  Merge/1 -> pedPreCollisionFlg/1
  If Action Subsystem1/1(CrossFlg) -> Merge/2
  If Action Subsystem/1(CrossFlg) -> Merge/1
  If/2 -> If Action Subsystem1/Action
  If/1 -> If Action Subsystem/Action
  Compare To Constant/1 -> If/1
  Abs/1 -> Compare To Constant/1
  VectorMulit/1(vectorMulit) -> Abs/1, If Action Subsystem1/1(vectorMulit)
  Bus Creator/1 -> Bus Selector/1, If Action Subsystem1/2(Coordinations)
  n2/1 -> Bus Creator/8  "n2"
  m2/1 -> Bus Creator/7  "m2"
  n1/1 -> Bus Creator/6  "n1"
  m1/1 -> Bus Creator/5  "m1"
  y2/1 -> Bus Creator/4  "y2"
  x2/1 -> Bus Creator/3  "x2"
  y1/1 -> Bus Creator/2  "y1"
  x1/1 -> Bus Creator/1  "x1"

== system TosAeb/FcwAebJa_Step_sys/ALG_AEBSystem/ALG_AEBController/AEB_LatObjInfoCalcApp/LatCycObjCalc/LatCycObjCalc/latCycObjInfoCalc/ObjCrossFlgCal/CycPreCollisionCalcFunc/PreRefPointEgoCalc ==
  subjVel : Inport
  tPredTime : Inport  Port=2  OutDataTypeStr=single
  subjLongAccel : Inport  Port=3
  subjLatAccel : Inport  Port=4
  subjYawRate : Inport  Port=5
  Add : Sum  OutDataTypeStr=single
  Gain : Gain  Gain=0.5  OutDataTypeStr=single
  Gain1 : Gain  Gain=0.5  OutDataTypeStr=single
  Math Function : Math  Operator=square  SignedPower=on  OutDataTypeStr=single  SaturateOnIntegerOverflow=on
  Product{,1..3} (4 alike) : Product  OutDataTypeStr=single
  " dxPreRefPoint" : Outport
  " dyPreRefPoint" : Outport  Port=2
  YawAnglePre : Outport  Port=3
  wires:
  Product1/1 -> Gain/1
  Product/1 -> Add/1
  subjVel/1 -> Product/1
  tPredTime/1 -> Product/2, Product3/1, Math Function/1
  Math Function/1 -> Product2/1, Product1/1
  subjLongAccel/1 -> Product1/2
  Add/1 -> " dxPreRefPoint"/1
  subjLatAccel/1 -> Product2/2
  Product2/1 -> Gain1/1
  subjYawRate/1 -> Product3/2
  Product3/1 -> YawAnglePre/1
  Gain/1 -> Add/2
  Gain1/1 -> " dyPreRefPoint"/1

== system TosAeb/FcwAebJa_Step_sys/ALG_AEBSystem/ALG_AEBController/AEB_LatObjInfoCalcApp/LatCycObjCalc/LatCycObjCalc/latCycObjInfoCalc/ObjCrossFlgCal/CycPreCollisionCalcFunc/SubjBoundingBox ==
  dxPreRefPoint : Inport  OutDataTypeStr=single
  dyPreRefPoint : Inport  Port=2  OutDataTypeStr=single
  sincosCoef : Inport  Port=3
  Abs : Abs
  Bus Creator : BusCreator  Inputs=6
  Constant : Constant  Value=P_Global_DxvFrontBumper_m
  Constant1 : Constant  Value=P_Global_DxvRearBumper_m
  Constant2 : Constant  Value=P_Global_VehicleWidthMin_m
  Mux : Mux
  Mux1 : Mux
  SubjLLCoordinate : Subsystem
  SubjLUCoordinate : Subsystem
  SubjRLCoordinate : Subsystem
  SubjRUCoordinate : Subsystem
  subjDxPre : Outport
  subjDyPre : Outport  Port=2
  wires:
  [dyPreRL] -> Mux1/4
  [dyPreLL] -> Mux1/3
  [dyPreLU] -> Mux1/2
  [dyPreRU] -> Mux1/1
  [dxPreRL] -> Mux/4
  [dxPreLL] -> Mux/3
  [dxPreLU] -> Mux/2
  [dxPreRU] -> Mux/1
  Mux1/1 -> subjDyPre/1
  Mux/1 -> subjDxPre/1
  SubjRLCoordinate/2(dyPreRL) -> [dyPreRL]  "dyPreRL"
  SubjRLCoordinate/1(dxPreRL) -> [dxPreRL]  "dxPreRL"
  SubjRUCoordinate/2(dyPreRU) -> [dyPreRU]  "dyPreRU"
  SubjRUCoordinate/1(dxPreRU) -> [dxPreRU]  "dxPreRU"
  SubjLLCoordinate/2(dyPreLL) -> [dyPreLL]  "dyPreLL"
  SubjLLCoordinate/1(dxPreLL) -> [dxPreLL]  "dxPreLL"
  SubjLUCoordinate/2(dyPreLU) -> [dyPreLU]  "dyPreLU"
  SubjLUCoordinate/1(dxPreLU) -> [dxPreLU]  "dxPreLU"
  Bus Creator/1 -> SubjLUCoordinate/1(inputSignals), SubjLLCoordinate/1(inputSignals), SubjRUCoordinate/1(inputSignals), SubjRLCoordinate/1(inputSignals)
  dxPreRefPoint/1 -> Bus Creator/1  "dxPreRefPoint"
  dyPreRefPoint/1 -> Bus Creator/2  "dyPreRefPoint"
  sincosCoef/1 -> Bus Creator/3  "sincosCoef"
  Constant/1 -> Bus Creator/4  "dxFrontBumperToRearAxis"
  Abs/1 -> Bus Creator/5  "dxRearBumperToRearAxis"
  Constant2/1 -> Bus Creator/6  "subjWidth"
  Constant1/1 -> Abs/1

== system TosAeb/FcwAebJa_Step_sys/ALG_AEBSystem/ALG_AEBController/AEB_LatObjInfoCalcApp/LatCycObjCalc/LatCycObjCalc/latCycObjInfoCalc/ObjCrossFlgCal/CycPreCollisionCalcFunc/sincosCoefCalc ==
  angle : Inport  OutDataTypeStr=single
  Bus Creator : BusCreator  Inputs=2
  Sincos : Trigonometry  Operator=sincos
  sincosCoef : Outport
  wires:
  Bus Creator/1 -> sincosCoef/1  "sincosCoef"
  Sincos/2 -> Bus Creator/2  "cosCoef"
  angle/1 -> Sincos/1
  Sincos/1 -> Bus Creator/1  "sinCoef"

== system TosAeb/FcwAebJa_Step_sys/ALG_AEBSystem/ALG_AEBController/AEB_LatObjInfoCalcApp/LatCycObjCalc/LatCycObjCalc/latCycObjInfoCalc/ObjCrossFlgCal/CycPreCollisionCalcFunc/sincosCoefCalc1 ==
  angle : Inport
  Bus Creator : BusCreator  Inputs=2
  Sincos : Trigonometry  Operator=sincos
  sincosCoef : Outport
  wires:
  Sincos/1 -> Bus Creator/1  "sinCoef"
  angle/1 -> Sincos/1
  Sincos/2 -> Bus Creator/2  "cosCoef"
  Bus Creator/1 -> sincosCoef/1  "sincosCoef"

== system TosAeb/FcwAebJa_Step_sys/ALG_AEBSystem/ALG_AEBController/AEB_LatObjInfoCalcApp/LatPedObjCalc/LatPedCollisonAssessInfo/LatPedCollisonAssessInfo/LatPedLongDistCondCalc/DistSecureCalc/If Action Subsystem ==
  subjVel : Inport
  Action Port : ActionPort  ActionPortLabel=else
  1-D Lookup Table : Lookup_n-D  NumberOfTableDimensions=1  BreakpointsForDimension1=P_AEB_LatPedCA_SubjVelDxSecureUnder40KphX  BeginIndexSearchUsingPreviousIndexResult=on  Table=P_AEB_LatPedCA_DxSecureY  ExtrapMethod=Clip  InternalRulePriority=Speed  RndMeth=Simplest  InputSameDT=on
  distSecure : Outport
  wires:
  subjVel/1 -> 1-D Lookup Table/1
  1-D Lookup Table/1 -> distSecure/1

== system TosAeb/FcwAebJa_Step_sys/ALG_AEBSystem/ALG_AEBController/AEB_LatObjInfoCalcApp/LatPedObjCalc/LatPedCollisonAssessInfo/LatPedCollisonAssessInfo/LatPedLongDistCondCalc/DistSecureCalc/If Action Subsystem1 ==
  Action Port : ActionPort  ActionPortLabel=if(u1)
  Constant : Constant  Value=CNNull
  distSecure : Outport
  wires:
  Constant/1 -> distSecure/1

== system TosAeb/FcwAebJa_Step_sys/ALG_AEBSystem/ALG_AEBController/AEB_LatObjInfoCalcApp/LatPedObjCalc/LatPedCollisonAssessInfo/LatPedCollisonAssessInfo/LatPedLongDistCondCalc/PedDistBrakeFullCalc/BrkSys ==
  subjVel : Inport
  subjLongAccel : Inport  Port=2  OutDataTypeStr=single
  AccelGredientMaxLookupTable : Lookup_n-D  NumberOfTableDimensions=1  BreakpointsForDimension1=P_AEB_LatPedCA_AccelGredientMaxX  Table=P_AEB_LatPedCA_AccelGredientMaxY  ExtrapMethod=Clip  InternalRulePriority=Speed  RndMeth=Simplest  InputSameDT=on
  DecelMaxLookupTable : Lookup_n-D  NumberOfTableDimensions=1  BreakpointsForDimension1=P_AEB_LatPedCA_DecelMaxX  Table=P_AEB_LatPedCA_DecelMaxY  ExtrapMethod=Clip  InternalRulePriority=Speed  RndMeth=Simplest  InputSameDT=on
  MinMax : MinMax  Function=max  Inputs=2  OutDataTypeStr=single
  P_LatPedCA_AccelGredientMax : Constant  Commented=on  Value=P_AEB_LatPedCA_AccelGredientMax  OutDataTypeStr=single
  P_LatPedCA_BrkDlyTime : Constant  Value=P_AEB_LatPedCA_BrkDlyTime  OutDataTypeStr=single
  P_LatPedCA_DecelMax : Constant  Commented=on  Value=P_AEB_LatPedCA_DecelMax  OutDataTypeStr=single
  P_LatPedCA_DecelMaxOver22Kph : Constant  Commented=on  Value=P_AEB_LatPedCA_DecelMaxOver22Kph  OutDataTypeStr=single
  P_LatPedCA_VelAccelMaxVelThres : simulink/Logic and Bit Operations/Compare To Constant  Commented=on  relop=>=  const=P_AEB_LatPedCA_VelAccelMaxVelThres  OutDataTypeStr=boolean
  P_LatPedCA_VelReduceMax : Constant  Value=P_AEB_LatPedCA_VelReduceMax  OutDataTypeStr=single
  P_LatPedCA_VelReduceMaxOver40Kph : Constant  Value=P_AEB_LatPedCA_VelReduceMaxOver40Kph  OutDataTypeStr=single
  P_LatPedCA_VelReduceMaxVelThres : simulink/Logic and Bit Operations/Compare To Constant  relop=>=  const=P_AEB_LatPedCA_VelReduceMaxVelThres  OutDataTypeStr=boolean
  Switch : Switch  Criteria=u2 ~= 0  OutDataTypeStr=single
  Switch1 : Switch  Commented=on  Criteria=u2 ~= 0  OutDataTypeStr=single
  tDelay : Outport
  Kjerk : Outport  Port=2
  velDelta : Outport  Port=3
  accelMax : Outport  Port=4
  subjLongAccelFilt : Outport  Port=5
  wires:
  P_LatPedCA_BrkDlyTime/1 -> tDelay/1
  P_LatPedCA_VelReduceMaxVelThres/1 -> Switch/2
  P_LatPedCA_VelReduceMaxOver40Kph/1 -> Switch/1
  P_LatPedCA_VelReduceMax/1 -> Switch/3
  AccelGredientMaxLookupTable/1 -> Kjerk/1
  Switch/1 -> velDelta/1
  DecelMaxLookupTable/1 -> MinMax/1, accelMax/1
  P_LatPedCA_DecelMaxOver22Kph/1 -> Switch1/3
  P_LatPedCA_DecelMax/1 -> Switch1/1
  P_LatPedCA_VelAccelMaxVelThres/1 -> Switch1/2
  subjVel/1 -> AccelGredientMaxLookupTable/1, DecelMaxLookupTable/1, P_LatPedCA_VelReduceMaxVelThres/1
  subjLongAccel/1 -> MinMax/2
  MinMax/1 -> subjLongAccelFilt/1

== system TosAeb/FcwAebJa_Step_sys/ALG_AEBSystem/ALG_AEBController/AEB_LatObjInfoCalcApp/LatPedObjCalc/LatPedCollisonAssessInfo/LatPedCollisonAssessInfo/LatPedLongDistCondCalc/PedDistBrakeFullCalc/DistPhase1Calc ==
  subjVel : Inport
  tPhase1 : Inport  Port=2  OutDataTypeStr=single
  subjLongAccelFilt : Inport  Port=3  OutDataTypeStr=single
  Add : Sum  OutDataTypeStr=single
  Constant : Constant  Value=0.5  OutDataTypeStr=single
  Product : Product  Inputs=4  OutDataTypeStr=single
  Product1 : Product  OutDataTypeStr=single
  distPhase1 : Outport
  wires:
  tPhase1/1 -> Product1/2, Product/2, Product/1
  subjLongAccelFilt/1 -> Product/3
  Constant/1 -> Product/4
  subjVel/1 -> Product1/1
  Product1/1 -> Add/1
  Product/1 -> Add/2
  Add/1 -> distPhase1/1

== system TosAeb/FcwAebJa_Step_sys/ALG_AEBSystem/ALG_AEBController/AEB_LatObjInfoCalcApp/LatPedObjCalc/LatPedCollisonAssessInfo/LatPedCollisonAssessInfo/LatPedLongDistCondCalc/PedDistBrakeFullCalc/DistPhase2Calc ==
  subjVel : Inport
  Kjerk : Inport  Port=2  OutDataTypeStr=single
  subjLongAccelFilt : Inport  Port=3  OutDataTypeStr=single
  accelMax : Inport  Port=4  OutDataTypeStr=single
  distPhase2Calc : Subsystem
  tPhase2Calc : Subsystem
  distPhase2 : Outport
  egoStopFlg : Outport  Port=2
  tJerkWork : Outport  Port=3
  tPhase2 : Outport  Port=4
  wires:
  subjLongAccelFilt/1 -> tPhase2Calc/3(subjLongAccelFilt), distPhase2Calc/2(subjAccelFilt)
  Kjerk/1 -> tPhase2Calc/2(Kjerk), distPhase2Calc/3(Kjerk)
  subjVel/1 -> distPhase2Calc/4(subjVel), tPhase2Calc/1(subjVel)
  accelMax/1 -> tPhase2Calc/4(accelMax)
  tPhase2Calc/3(tPhase2) -> tPhase2/1, distPhase2Calc/1(tPhase2)
  distPhase2Calc/1(distPhase2) -> distPhase2/1
  tPhase2Calc/2(tJerkWork) -> tJerkWork/1
  tPhase2Calc/1(egoStopFlg) -> egoStopFlg/1

== system TosAeb/FcwAebJa_Step_sys/ALG_AEBSystem/ALG_AEBController/AEB_LatObjInfoCalcApp/LatPedObjCalc/LatPedCollisonAssessInfo/LatPedCollisonAssessInfo/LatPedLongDistCondCalc/PedDistBrakeFullCalc/DistPhase3Calc ==
  egoStopFlg : Inport
  tJerkWork : Inport  Port=2  OutDataTypeStr=single
  tPhase2 : Inport  Port=3  OutDataTypeStr=single
  tPhase1 : Inport  Port=4  OutDataTypeStr=single
  velDelta : Inport  Port=5  OutDataTypeStr=single
  subjVel : Inport  Port=6
  subjLongAccelFilt : Inport  Port=7  OutDataTypeStr=single
  Kjerk : Inport  Port=8  OutDataTypeStr=single
  accelMax : Inport  Port=9  OutDataTypeStr=single
  Bus Creator : BusCreator  Inputs=8
  Bus Selector : BusSelector  OutputSignals=subjVel,subjLongAccelFilt,tJerkWork,Kjerk,accelMax,velDelta
  Bus Selector1 : BusSelector  OutputSignals=subjVel,subjLongAccelFilt,Kjerk,tPhase1,tPhase2,accelMax
  If : If  IfExpression=u1
  Merge : Merge
  distPhase3Calc : Subsystem
  tPhase3Calc : Subsystem  TreatAsAtomicUnit=on
  tPhase3Calc1 : Subsystem  TreatAsAtomicUnit=on
  distPhase3 : Outport
  wires:
  If/1 -> tPhase3Calc1/Action
  egoStopFlg/1 -> If/1
  If/2 -> tPhase3Calc/Action
  tPhase3Calc1/1(tPhase3) -> Merge/1
  tPhase3Calc/1(tPhase3) -> Merge/2
  Merge/1 -> distPhase3Calc/1(tPhase3)
  distPhase3Calc/1(distPhase3) -> distPhase3/1
  accelMax/1 -> Bus Creator/8  "accelMax"
  Kjerk/1 -> Bus Creator/7  "Kjerk"
  subjLongAccelFilt/1 -> Bus Creator/6  "subjLongAccelFilt"
  subjVel/1 -> Bus Creator/5  "subjVel"
  tJerkWork/1 -> Bus Creator/1  "tJerkWork"
  tPhase1/1 -> Bus Creator/2  "tPhase1"
  tPhase2/1 -> Bus Creator/3  "tPhase2"
  velDelta/1 -> Bus Creator/4  "velDelta"
  Bus Creator/1 -> Bus Selector1/1, Bus Selector/1
  Bus Selector/3 -> tPhase3Calc/3(tJerkWork)  "<tJerkWork>"
  Bus Selector/4 -> tPhase3Calc/4(Kjerk)  "<Kjerk>"
  Bus Selector/2 -> tPhase3Calc/2(subjLongAccelFilt)  "<subjLongAccelFilt>"
  Bus Selector/5 -> tPhase3Calc/5(accelMax)  "<accelMax>"
  Bus Selector/1 -> tPhase3Calc/1(subjVel)  "<subjVel>"
  Bus Selector/6 -> tPhase3Calc/6(velDelta)  "<velDelta>"
  Bus Selector1/6 -> distPhase3Calc/7(accelMax)  "<accelMax>"
  Bus Selector1/5 -> distPhase3Calc/6(tPhase2)  "<tPhase2>"
  Bus Selector1/4 -> distPhase3Calc/5(tPhase1)  "<tPhase1>"
  Bus Selector1/3 -> distPhase3Calc/4(Kjerk)  "<Kjerk>"
  Bus Selector1/2 -> distPhase3Calc/3(subjLongAccelFilt)  "<subjLongAccelFilt>"
  Bus Selector1/1 -> distPhase3Calc/2(subjVel)  "<subjVel>"

== system TosAeb/FcwAebJa_Step_sys/ALG_AEBSystem/ALG_AEBController/AEB_LatObjInfoCalcApp/LatPedObjCalc/LatPedCollisonAssessInfo/LatPedCollisonAssessInfo/LatPedLongDistCondCalc/PedDistBrakeFullCalc/distBrakeCalc ==
  distPhase1 : Inport  OutDataTypeStr=single
  distPhase2 : Inport  Port=2  OutDataTypeStr=single
  distPhase3 : Inport  Port=3  OutDataTypeStr=single
  Add : Sum  Inputs=3  OutDataTypeStr=single
  distBrake : Outport
  wires:
  distPhase2/1 -> Add/2
  distPhase1/1 -> Add/1
  distPhase3/1 -> Add/3
  Add/1 -> distBrake/1

== system TosAeb/FcwAebJa_Step_sys/ALG_AEBSystem/ALG_AEBController/AEB_LatObjInfoCalcApp/LatPedObjCalc/LatPedObjCalc/latPedObjInfoCalc/ObjColiisionRiskProbCalc/CollisionRiskProbCalc/CollisionRiskProbCalc ==
  dxReginEgoRUCorr : Inport  OutDataTypeStr=single
  dyReginEgoRUCorr : Inport  Port=2  OutDataTypeStr=single
  dyReginEgoLLCorr : Inport  Port=3  OutDataTypeStr=single
  dxReginEgoLLCorr : Inport  Port=4  OutDataTypeStr=single
  dxReginObjRUCorr : Inport  Port=5  OutDataTypeStr=single
  dyReginObjRUCorr : Inport  Port=6  OutDataTypeStr=single
  dxReginObjLLCorr : Inport  Port=7  OutDataTypeStr=single
  dyReginObjLLCorr : Inport  Port=8  OutDataTypeStr=single
  Bus Creator : BusCreator  Inputs=8
  Bus Selector : BusSelector  OutputSignals=dyReginEgoRUCorr,dyReginEgoLLCorr,dyReginObjRUCorr,dyReginObjLLCorr
  Bus Selector1 : BusSelector  OutputSignals=dxReginEgoRUCorr,dxReginEgoLLCorr,dxReginObjRUCorr,dxReginObjLLCorr
  Bus Selector2 : BusSelector  OutputSignals=dxReginObjLLCorr,dyReginObjRUCorr,dyReginObjLLCorr
  CriticalityPointCalc : Subsystem
  dxOverlapFlow : Stateflow chart  TreatAsAtomicUnit=on
  dyOverlapFlow : Stateflow chart  TreatAsAtomicUnit=on
  pedCollisionRiskProbCalc : Subsystem
  dxPreCollison : Outport
  dyPreCollison : Outport  Port=2
  collisionRiskProb : Outport  Port=3
  dyCollisionRiskProb : Outport  Port=4
  dxCollisionRiskProb : Outport  Port=5
  wires:
  dyOverlapFlow/1(dyOverlap) -> pedCollisionRiskProbCalc/1(dyOverlap)
  dyOverlapFlow/2(dyOverlapLimit) -> pedCollisionRiskProbCalc/2(dyOverlapLimit)
  dxOverlapFlow/1(dxOverlap) -> pedCollisionRiskProbCalc/3(dxOverlap)
  dxOverlapFlow/2(dxOverlapLimit) -> pedCollisionRiskProbCalc/4(dxOverlapLimit)
  pedCollisionRiskProbCalc/1(collisionRiskProb) -> collisionRiskProb/1
  dyReginEgoLLCorr/1 -> Bus Creator/3  "dyReginEgoLLCorr"
  dyReginEgoRUCorr/1 -> Bus Creator/2  "dyReginEgoRUCorr"
  dxReginEgoLLCorr/1 -> Bus Creator/4  "dxReginEgoLLCorr"
  dxReginObjRUCorr/1 -> Bus Creator/5  "dxReginObjRUCorr"
  dxReginEgoRUCorr/1 -> Bus Creator/1  "dxReginEgoRUCorr"
  dyReginObjRUCorr/1 -> Bus Creator/6  "dyReginObjRUCorr"
  dxReginObjLLCorr/1 -> Bus Creator/7  "dxReginObjLLCorr"
  dyReginObjLLCorr/1 -> Bus Creator/8  "dyReginObjLLCorr"
  Bus Creator/1 -> Bus Selector1/1, Bus Selector/1, Bus Selector2/1
  Bus Selector/1 -> dyOverlapFlow/1(dyReginEgoRUCorr)  "<dyReginEgoRUCorr>"
  Bus Selector/2 -> dyOverlapFlow/2(dyReginEgoLLCorr)  "<dyReginEgoLLCorr>"
  Bus Selector1/1 -> dxOverlapFlow/1(dxReginEgoRUCorr)  "<dxReginEgoRUCorr>"
  Bus Selector1/2 -> dxOverlapFlow/2(dxReginEgoLLCorr)  "<dxReginEgoLLCorr>"
  Bus Selector2/1 -> CriticalityPointCalc/1(dxReginObjLLCorr)  "<dxReginObjLLCorr>"
  Bus Selector2/2 -> CriticalityPointCalc/2(dyReginObjRUCorr)  "<dyReginObjRUCorr>"
  Bus Selector2/3 -> CriticalityPointCalc/3(dyReginObjLLCorr)  "<dyReginObjLLCorr>"
  CriticalityPointCalc/1(dxPreCollison) -> dxPreCollison/1
  CriticalityPointCalc/2(dyPreCollison) -> dyPreCollison/1
  Bus Selector/3 -> dyOverlapFlow/3(dyReginObjRUCorr)  "<dyReginObjRUCorr>"
  Bus Selector/4 -> dyOverlapFlow/4(dyReginObjLLCorr)  "<dyReginObjLLCorr>"
  Bus Selector1/3 -> dxOverlapFlow/3(dxReginObjRUCorr)  "<dxReginObjRUCorr>"
  Bus Selector1/4 -> dxOverlapFlow/4(dxReginObjLLCorr)  "<dxReginObjLLCorr>"
  pedCollisionRiskProbCalc/2(dyCollisionRiskProb) -> dyCollisionRiskProb/1
  pedCollisionRiskProbCalc/3(dxCollisionRiskProb) -> dxCollisionRiskProb/1

== system TosAeb/FcwAebJa_Step_sys/ALG_AEBSystem/ALG_AEBController/AEB_LatObjInfoCalcApp/LatPedObjCalc/LatPedObjCalc/latPedObjInfoCalc/ObjColiisionRiskProbCalc/CollisionRiskProbCalc/EgoReginCalc ==
  tPredTime : Inport  OutDataTypeStr=single
  subjVel : Inport  Port=2
  Abs : Abs
  Add : Sum  OutDataTypeStr=single
  Add1 : Sum  Inputs=+-  OutDataTypeStr=single
  Constant : Constant  Value=P_Global_DxvRearBumper_m
  Constant1 : Constant  Value=P_Global_VehicleWidthMin_m
  Constant2 : Constant  Value=P_Global_DxvFrontBumper_m
  Gain : Gain  Gain=-0.5
  Gain1 : Gain  Gain=0.5
  Product : Product  OutDataTypeStr=single
  dxReginEgoRU : Outport
  dyReginEgoRU : Outport  Port=2
  dxReginEgoLL : Outport  Port=3
  dyReginEgoLL : Outport  Port=4
  wires:
  Product/1 -> Add/1, Add1/1
  Constant2/1 -> Add/2
  Add/1 -> dxReginEgoRU/1
  Constant1/1 -> Gain1/1, Gain/1
  Gain/1 -> dyReginEgoRU/1
  Gain1/1 -> dyReginEgoLL/1
  Constant/1 -> Abs/1
  Add1/1 -> dxReginEgoLL/1
  tPredTime/1 -> Product/1
  subjVel/1 -> Product/2
  Abs/1 -> Add1/2
